simulink model "Complete_Model"  (R2023a) (continued)
== system Complete_Model/Equivalent Wind Park/300MW Eq WT3/big scope == (continued)
  To Workspace4 : ToWorkspace  VariableName=Wind_speed10  SaveFormat=Timeseries  SampleTime=-1
  To Workspace5 : ToWorkspace  VariableName=omega_m10  SaveFormat=Timeseries  SampleTime=-1
  To Workspace6 : ToWorkspace  VariableName=omega_m_opt10  SaveFormat=Timeseries  SampleTime=-1
  To Workspace7 : ToWorkspace  VariableName=omega_m_sync10  SaveFormat=Timeseries  SampleTime=-1
  To Workspace8 : ToWorkspace  VariableName=lambda10  SaveFormat=Timeseries  SampleTime=-1
  To Workspace9 : ToWorkspace  VariableName=iqr_ref10  SaveFormat=Timeseries  SampleTime=-1
  wires:
  [vqg10] -> To Workspace32/1  "vqg_mes"
  [vqg_ref10] -> To Workspace31/1  "vqg_ref"
  [iqg10] -> To Workspace30/1  "iqg_mes"
  [iqg_ref10] -> To Workspace29/1  "iqg_ref"
  [Qg_mes10] -> To Workspace28/1  "Qg_mes"
  [vdg10] -> To Workspace26/1  "vdg_mes"
  [Qg_ref10] -> To Workspace27/1  "Qg_ref"
  [vdg_ref10] -> To Workspace25/1  "vdg_ref"
  [idg10] -> To Workspace24/1  "idg_mes"
  [idg_ref10] -> To Workspace23/1  "idg_ref"
  [Vbus_mes10] -> To Workspace22/1  "Vbus_mes"
  [Vbus_ref10] -> To Workspace21/1  "Vbus_ref"
  [Ig10] -> To Workspace20/1  "Ig"
  [Ir_mes10] -> To Workspace19/1  "Ir"
  [Iout10] -> To Workspace18/1  "Iout"
  [Vout10] -> To Workspace17/1  "Vout"
  [vqr_ref10] -> To Workspace16/1  "vqr_ref"
  [idr10] -> To Workspace15/1  "idr"
  [idr_ref10] -> To Workspace14/1  "idr_ref"
  [Tr10] -> To Workspace13/1  "Tr"
  [Tr_ref10] -> To Workspace12/1  "Tr_ref_MPPT"
  [vdr_ref10] -> To Workspace11/1  "vdr_ref"
  [iqr10] -> To Workspace10/1  "iqr"
  [iqr_ref10] -> To Workspace9/1  "iqr_ref"
  [lambda10] -> To Workspace8/1  "Tip-Speed Ratio"
  [P_m10] -> To Workspace/1  "P meca"
  [omega_m10] -> To Workspace5/1  "omega_m"
  [v_wind10] -> To Workspace4/1  "Wind speed"
  [lambda10] -> External Quantities/4  "Tip-Speed Ratio"
  Gain3/1 -> Mux6/1, To Workspace6/1  "omega_m,opt"
  Divide3/1 -> Gain3/1
  Constant1/1 -> Divide3/3
  Constant2/1 -> Divide3/2
  [v_wind10] -> Divide3/1
  [idr10] -> Mux3/2  "idr"
  [Tr10] -> Mux2/2  "Tr"
  [vdr_ref10] -> Rotor Side/3  "vdr_ref"
  [iqr_ref10] -> Mux1/1  "iqr_ref"
  Mux13/1 -> Grid Side/4
  [Qg_mes10] -> Mux13/2  "Qg_mes"
  [Qg_ref10] -> Mux13/1  "Qg_ref"
  Mux12/1 -> Grid Side/6
  [vqg_ref10] -> Mux12/1  "vqg_ref"
  [vqg10] -> Mux12/2  "vqg_mes"
  Gain2/1 -> Mux9/4, To Workspace3/1  "P rotor"
  [Pout10] -> Gain6/1
  Mux9/1 -> Power/1
  [P_m10] -> Mux9/1  "P meca"
  [Pr_mes_machine10] -> Gain2/1
  Gain7/1 -> Mux9/3, To Workspace2/1  "P stator"
  [Ps_mes_machine10] -> Gain7/1
  Gain6/1 -> Mux9/2, To Workspace1/1  "P out"
  Mux11/1 -> Grid Side/3
  [vdg10] -> Mux11/2  "vdg_mes"
  [vdg_ref10] -> Mux11/1  "vdg_ref"
  [P_m10] -> Mux8/1  "P_mech"
  Constant/1 -> Mux6/3, To Workspace7/1  "synchronous rotor speed"
  [omega_m10] -> Mux6/2  "omega_m"
  [v_wind10] -> External Quantities/1  "Wind speed"
  Mux6/1 -> External Quantities/2
  Mux8/1 -> External Quantities/3
  [Pout10] -> Gain/1
  Gain/1 -> Mux8/2  "P_grid"
  [omega_m10] -> Rotor Side/1  "omega_m"
  [idg_ref10] -> Mux5/1  "idg_ref"
  Mux4/1 -> Grid Side/1
  [idg10] -> Mux5/2  "idg_mes"
  Mux5/1 -> Grid Side/2
  [Vbus_mes10] -> Mux4/2  "Vbus_mes"
  Mux7/1 -> Grid Side/5
  [Vbus_ref10] -> Mux4/1  "Vbus_ref"
  [iqg10] -> Mux7/2  "iqg_mes"
  [Ir_mes10] -> Grid Side/9  "Ir"
  [Vout10] -> Grid Side/7  "Vout"
  [iqg_ref10] -> Mux7/1  "iqg_ref"
  [Ig10] -> Grid Side/8  "Ig"
  [Ir_mes10] -> Rotor Side/9  "Ir"
  [Iout10] -> Rotor Side/8  "Iout"
  [Vout10] -> Rotor Side/7  "Vout"
  [vqr_ref10] -> Rotor Side/6  "vqr_ref"
  [idr_ref10] -> Mux3/1  "idr_ref"
  Mux3/1 -> Rotor Side/5
  Mux2/1 -> Rotor Side/4
  [Tr_ref10] -> Mux2/1  "Tr_ref_MPPT"
  Mux1/1 -> Rotor Side/2
  [iqr10] -> Mux1/2  "iqr"

== system Complete_Model/Equivalent Wind Park/300MW Eq WT4/Aerodynamic Model ==
  note: Gearbox ratio
  note: Motor convention : Generated power
      |  is negative
  note: \Omega_m blades
  note: Cp
  note: \lambda = \Omega_m R / v_{wind}
  note: C_p (\lambda)
  note: v_{wind}
  Vw : Inport
  C_p (\lambda) : Lookup_n-D  NumberOfTableDimensions=1  BreakpointsForDimension1=tab_lambda  Table=Cp  InternalRulePriority=Speed  RndMeth=Simplest
  Constant : Constant  Value=Radius
  Divide : Product  Inputs=*/
  Divide1 : Product  Inputs=**/
  Fcn2 : Fcn  Expr=0.5*rho*pi*Radius^2*u(2)^3*u(1)
  Gain : Gain  Gain=1/N
  Gain2 : Gain  Gain=n_wt_agg
  Gain4 : Gain  Gain=-1
  Mux2 : Mux  Inputs=2
  Tm : Outport
  tag scope: P_m9=global, Tm9=global, lambda9=global
  wires:
  Gain4/1 -> Divide/1
  Constant/1 -> Divide1/1
  Divide/1 -> Tm/1, [Tm9]
  [omega_m9] -> Divide/2
  Gain2/1 -> Gain4/1, [P_m9]
  Fcn2/1 -> Gain2/1
  Mux2/1 -> Fcn2/1
  C_p (\lambda)/1 -> Mux2/1
  Divide1/1 -> [lambda9], C_p (\lambda)/1  "lambda"
  Vw/1 -> Mux2/2, Divide1/3
  Gain/1 -> Divide1/2
  [omega_m9] -> Gain/1

== system Complete_Model/Equivalent Wind Park/300MW Eq WT4/Grid Side Control ==
  Vbus_ref : Inport
  Qg_ref : Inport  Port=2
  Vbus : Inport  Port=3
  Ig_mes : Inport  Port=4
  Vs_mes : Inport  Port=5
  Demux : Demux  Outputs=2
  Demux1 : Demux  Outputs=2
  Gain : Gain  Gain=Kqg
  Gain1 : Gain  Gain=Kpg
  Normalized for PWM : Gain  Gain=1/(Vbus/2)
  "PI " : Subsystem
  PI id : Subsystem
  PI iq : Subsystem
  Sum : Sum  Inputs=|++
  Vs_mes1 : InportShadow  Port=5
  abc->alpha/beta : Subsystem
  abc->alpha/beta1 : Subsystem
  alpha/beta->abc : Subsystem
  alpha/beta->d/q : Subsystem
  alpha/beta->d/q1 : Subsystem
  angle2 : Subsystem
  cancellation of coupling terms : Subsystem
  d/q->alpha/beta : Subsystem
  Vabc_ref : Outport
  tag scope: Qg_ref9=global, Vbus_ref9=global, idg9=global, idg_ref9=global, iqg9=global, iqg_ref9=global, vdg9=global, vqg9=global
  wires:
  Vs_mes1/1 -> Sum/2
  angle2/1(theta_g) -> [theta_grid9]
  [theta_grid9] -> alpha/beta->d/q1/2(theta)
  [theta_grid9] -> d/q->alpha/beta/2(theta)
  [theta_grid9] -> alpha/beta->d/q/2(theta)
  Demux/2 -> PI iq/2(meas), [iqg9]
  Demux/1 -> PI id/2(meas), [idg9]
  Vbus/1 -> "PI "/2(meas)
  Demux1/1 -> [vdg9]
  Demux1/2 -> [vqg9]
  alpha/beta->d/q1/1(d/q) -> Demux1/1
  abc->alpha/beta1/1(alpha/beta) -> alpha/beta->d/q1/1(D/Q)
  [Vg9] -> abc->alpha/beta1/1(abc)
  cancellation of coupling terms/1(out) -> d/q->alpha/beta/1(d/q)
  Sum/1 -> Normalized for PWM/1
  alpha/beta->abc/1(abc) -> Sum/1
  Qg_ref/1 -> Gain/1, [Qg_ref9]
  Gain1/1 -> PI id/1(ref), [idg_ref9]  "idg"
  "PI "/1(out) -> Gain1/1  "Pg_ref"
  Gain/1 -> [iqg_ref9], PI iq/1(ref)  "iqg"
  Vbus_ref/1 -> "PI "/1(ref), [Vbus_ref9]
  alpha/beta->d/q/1(d/q) -> Demux/1
  PI iq/1(out) -> cancellation of coupling terms/2(Vq*)
  PI id/1(out) -> cancellation of coupling terms/1(Vd*)
  Vs_mes/1 -> angle2/1(Vs_mes)
  Normalized for PWM/1 -> Vabc_ref/1
  abc->alpha/beta/1(alpha/beta) -> alpha/beta->d/q/1(D/Q)
  Ig_mes/1 -> abc->alpha/beta/1(abc)
  d/q->alpha/beta/1(D/Q) -> alpha/beta->abc/1(D/Q)

== system Complete_Model/Equivalent Wind Park/300MW Eq WT4/Power Calculations ==
  Demux{,1..3} (4 alike) : Demux  Outputs=2
  Divide : Product  Inputs=**
  Gain : Gain  Gain=3/2
  Gain1 : Gain  Gain=-1
  Gain2 : Gain  Gain=-1
  Power (3ph, Instantaneous){1..4} (4 alike) : spsPower3phInstantaneousLib/Power (3ph, Instantaneous)
  Scope{1..8,10..13} (12 alike) : Scope
  Scope9 : Scope  NumInputPorts=2
  "Second-Order " : spsSecondOrderFilterLib/Second-Order Filter  FilterType=Lowpass  Fo=17  Zeta=0.7  Ts=7.4074e-05  Initialize=off  Vac_Init="[0,  0,  60]"  Vdc_Init=0  PlotResponse=off  FreqRange=[0, 500, 1]
      settings: AttributesFormatString=f0=%<Fo>
  Second-Order 1 : spsSecondOrderFilterLib/Second-Order Filter  FilterType=Lowpass  Fo=17  Zeta=0.7  Ts=7.4074e-05  Initialize=off  Vac_Init="[0,  0,  60]"  Vdc_Init=0  PlotResponse=off  FreqRange=[0, 500, 1]
      settings: AttributesFormatString=f0=%<Fo>
  Second-Order Low-Pass Filter {1..7} (7 alike) : spsSecondOrderFilterLib/Second-Order Filter  FilterType=Lowpass  Fo=17  Zeta=0.7  Ts=7.4074e-05  Initialize=off  Vac_Init="[0,  0,  60]"  Vdc_Init=0  PlotResponse=off  FreqRange=[0, 500, 1]
      settings: AttributesFormatString=f0=%<Fo>
  Subsystem : Subsystem
  Subsystem1 : Subsystem
  Subsystem2 : Subsystem
  Subsystem3 : Subsystem
  To Workspace1 : ToWorkspace  VariableName=Iout_d9  SaveFormat=Timeseries  SampleTime=-1
  To Workspace2 : ToWorkspace  VariableName=Ig_d9  SaveFormat=Timeseries  SampleTime=-1
  To Workspace21 : ToWorkspace  VariableName=Vout_d9  SaveFormat=Timeseries  SampleTime=-1
  To Workspace3 : ToWorkspace  VariableName=Vg_d9  SaveFormat=Timeseries  SampleTime=-1
  alpha/beta->d/q : Subsystem
  alpha/beta->d/q1 : Subsystem
  alpha/beta->d/q2 : Subsystem
  alpha/beta->d/q3 : Subsystem
  angle1 : Subsystem
  angle2 : Subsystem
  tag scope: Pg_mes9=global, Pout9=global, Pr_mes_machine9=global, Ps_mes_machine9=global, Qg_mes9=global, Qout9=global, Qr_mes_machine9=global, Qs_mes_machine9=global
  wires:
  [Vg9] -> angle1/1(Vs_mes), Subsystem2/1(abc)
  Subsystem2/1(alpha/beta) -> alpha/beta->d/q2/1(D/Q)
  alpha/beta->d/q2/1(d/q) -> Demux2/1
  Demux2/1 -> To Workspace3/1, Scope10/1
  Demux2/2 -> Scope11/1
  Demux3/2 -> Scope13/1
  Demux3/1 -> Scope12/1, To Workspace2/1
  alpha/beta->d/q3/1(d/q) -> Demux3/1
  Subsystem3/1(alpha/beta) -> alpha/beta->d/q3/1(D/Q)
  angle1/1(theta_g) -> alpha/beta->d/q3/2(theta), alpha/beta->d/q2/2(theta)
  [Ig9] -> Subsystem3/1(abc)
  Gain2/1 -> Second-Order 1/1
  Gain/1 -> Gain2/1
  Gain1/1 -> Scope9/2
  Second-Order 1/1 -> Scope9/1  "Pout"
  Divide/1 -> Gain/1
  [Iout9] -> Subsystem1/1(abc)
  angle2/1(theta_g) -> alpha/beta->d/q/2(theta), alpha/beta->d/q1/2(theta)
  Subsystem1/1(alpha/beta) -> alpha/beta->d/q1/1(D/Q)
  alpha/beta->d/q1/1(d/q) -> Demux1/1
  Demux1/1 -> To Workspace1/1, Scope7/1, Divide/2
  Demux1/2 -> Scope8/1
  Demux/2 -> Scope6/1
  Demux/1 -> Scope2/1, To Workspace21/1, Divide/1
  alpha/beta->d/q/1(d/q) -> Demux/1
  Subsystem/1(alpha/beta) -> alpha/beta->d/q/1(D/Q)
  [Vout9] -> Subsystem/1(abc), angle2/1(Vs_mes)
  [Vr_mes9] -> "Power (3ph, Instantaneous)4"/1
  "Power (3ph, Instantaneous)1"/1 -> Second-Order Low-Pass Filter 7/1  "Pr"
  "Power (3ph, Instantaneous)4"/1 -> Second-Order Low-Pass Filter 5/1  "Pr"
  [Vout9] -> "Power (3ph, Instantaneous)2"/1
  "Power (3ph, Instantaneous)3"/2 -> Second-Order Low-Pass Filter 2/1
  [Vg9] -> "Power (3ph, Instantaneous)1"/1
  Second-Order Low-Pass Filter 2/1 -> [Qs_mes_machine9]  "Q_stator"
  [Vs_mes_machine9] -> "Power (3ph, Instantaneous)3"/1
  "Power (3ph, Instantaneous)2"/1 -> "Second-Order "/1
  Second-Order Low-Pass Filter 5/1 -> [Pr_mes_machine9]  "P_rotor"
  [Is_mes_machine9] -> "Power (3ph, Instantaneous)3"/2, Scope4/1
  Second-Order Low-Pass Filter 7/1 -> [Pg_mes9]  "P_rotor"
  [Ir_mes9] -> "Power (3ph, Instantaneous)4"/2, Scope5/1
  "Second-Order "/1 -> [Pout9], Gain1/1  "Pout"
  Second-Order Low-Pass Filter 6/1 -> [Qg_mes9]  "Q_rotor"
  Second-Order Low-Pass Filter 4/1 -> [Qr_mes_machine9]  "Q_rotor"
  "Power (3ph, Instantaneous)3"/1 -> Second-Order Low-Pass Filter 3/1  "Ps"
  [Ig9] -> Scope1/1, "Power (3ph, Instantaneous)1"/2
  "Power (3ph, Instantaneous)4"/2 -> Second-Order Low-Pass Filter 4/1
  [Iout9] -> "Power (3ph, Instantaneous)2"/2, Scope3/1
  "Power (3ph, Instantaneous)1"/2 -> Second-Order Low-Pass Filter 6/1
  Second-Order Low-Pass Filter 3/1 -> [Ps_mes_machine9]  "P_stator"
  Second-Order Low-Pass Filter 1/1 -> [Qout9]  "Qout"
  "Power (3ph, Instantaneous)2"/2 -> Second-Order Low-Pass Filter 1/1

== system Complete_Model/Equivalent Wind Park/300MW Eq WT4/Rotor Side Control ==
  note: Stator referred
  idr_ref : Inport
  Ir_mes : Inport  Port=2
  Vs_mes : Inport  Port=3
  theta_m_mes : Inport  Port=4
  speed_mes : Inport  Port=5
  D/Q->abc : Subsystem
  D/Q->d/q : Subsystem
  Demux : Demux  Outputs=2
  Gain : Gain  Gain=1/(-1.5*p*(Lm/Ls)*Fs)
  Gain1 : Gain  Gain=1/u
  Gain2 : Gain  Gain=1/u
  MPPT : Subsystem
  Normalized for PWM : Gain  Gain=1/(Vbus/2)
  PI id : Subsystem
  PI iq : Subsystem
  abc->alpha/beta : Subsystem
  angle : Subsystem
  cancellation of coupling terms : Subsystem
  d/q->D/Q : Subsystem
  Vabc_ref : Outport
  tag scope: Tr_ref9=global, idr9=global, idr_ref9=global, iqr9=global, iqr_ref9=global, vrabc_ref9=global
  wires:
  MPPT/1(Tm,opt) -> Gain/1, [Tr_ref9]
  d/q->D/Q/1(D/Q) -> D/Q->abc/1(D/Q)
  abc->alpha/beta/1(alpha/beta) -> D/Q->d/q/1(D/Q)
  Gain2/1 -> [vrabc_ref9], Normalized for PWM/1  "Rotor referred"
  D/Q->abc/1(abc) -> Gain2/1
  Normalized for PWM/1 -> Vabc_ref/1
  Gain1/1 -> abc->alpha/beta/1(abc)
  idr_ref/1 -> PI id/1(ref), [idr_ref9]
  Gain/1 -> PI iq/1(ref), [iqr_ref9]
  Demux/2 -> PI iq/2(meas), [iqr9]
  Demux/1 -> [idr9], PI id/2(meas)
  D/Q->d/q/1(d/q) -> Demux/1  "i_dq = rotor ref frame"
  speed_mes/1 -> MPPT/1(omega_m), cancellation of coupling terms/3(omega_m)
  cancellation of coupling terms/1(out) -> d/q->D/Q/1(d/q)  "Vdr_ref,Vqr_ref"
  PI iq/1(out) -> cancellation of coupling terms/2(Vq*)
  PI id/1(out) -> cancellation of coupling terms/1(Vd*)
  angle/1(theta_r) -> D/Q->d/q/2(theta), d/q->D/Q/2(theta)
  Vs_mes/1 -> angle/1(Vs_mes)
  theta_m_mes/1 -> angle/2(theta_m)
  Ir_mes/1 -> Gain1/1

== system Complete_Model/Equivalent Wind Park/300MW Eq WT4/Subsystem ==
  In1 : Inport
  Bus Selector : BusSelector  OutputSignals=Mechanical.Rotor angle thetam (rad),Mechanical.Rotor speed (wm),Mechanical.Electromagnetic torque Te (N*m)
  Second-Order Low-Pass Filter 3 : spsSecondOrderFilterLib/Second-Order Filter  FilterType=Lowpass  Fo=17  Zeta=0.7  Ts=7.4074e-05  Initialize=off  Vac_Init="[0,  0,  60]"  Vdc_Init=0  PlotResponse=off  FreqRange=[0, 500, 1]
      settings: AttributesFormatString=f0=%<Fo>
  tag scope: Tr9=global, omega_m9=global, theta9=global
  wires:
  Second-Order Low-Pass Filter 3/1 -> [omega_m9]
  Bus Selector/3 -> [Tr9]  "<Electromagnetic torque Te (N*m)>"
  Bus Selector/1 -> [theta9]  "<Rotor angle thetam (rad)>"
  In1/1 -> Bus Selector/1
  Bus Selector/2 -> Second-Order Low-Pass Filter 3/1  "<Rotor speed (wm)>"

== system Complete_Model/Equivalent Wind Park/300MW Eq WT4/Subsystem1 ==
  Constant4 : Constant  Value=0
  Step : Step  Time=50  After=-0.2*Ps  SampleTime=0
  Sum14 : Sum  Inputs=++|
  Out1 : Outport
  wires:
  Sum14/1 -> Out1/1
  Step/1 -> Sum14/1
  Constant4/1 -> Sum14/2

== system Complete_Model/Equivalent Wind Park/300MW Eq WT4/Subsystem2 ==
  Constant1 : Constant  Value=9
  Ramp1 : simulink/Sources/Ramp  slope=-0.4  start=13  InitialOutput=0
  Ramp2 : simulink/Sources/Ramp  slope=0.4  start=8  InitialOutput=0
  Sum1 : Sum  Inputs=++|
  Sum2 : Sum  Inputs=++|
  v_wind : Outport
  tag scope: v_wind9=global
  wires:
  Constant1/1 -> Sum2/2
  Sum2/1 -> v_wind/1, [v_wind9]
  Ramp1/1 -> Sum1/2
  Sum1/1 -> Sum2/1
  Ramp2/1 -> Sum1/1

== system Complete_Model/Equivalent Wind Park/300MW Eq WT4/Subsystem8 ==
  Constant4 : Constant  Value=0
  Step : Step  Time=55  After=Idr_rated*0.2  SampleTime=0
  Sum14 : Sum  Inputs=++|
  Out1 : Outport
  wires:
  Sum14/1 -> Out1/1
  Constant4/1 -> Sum14/2
  Step/1 -> Sum14/1

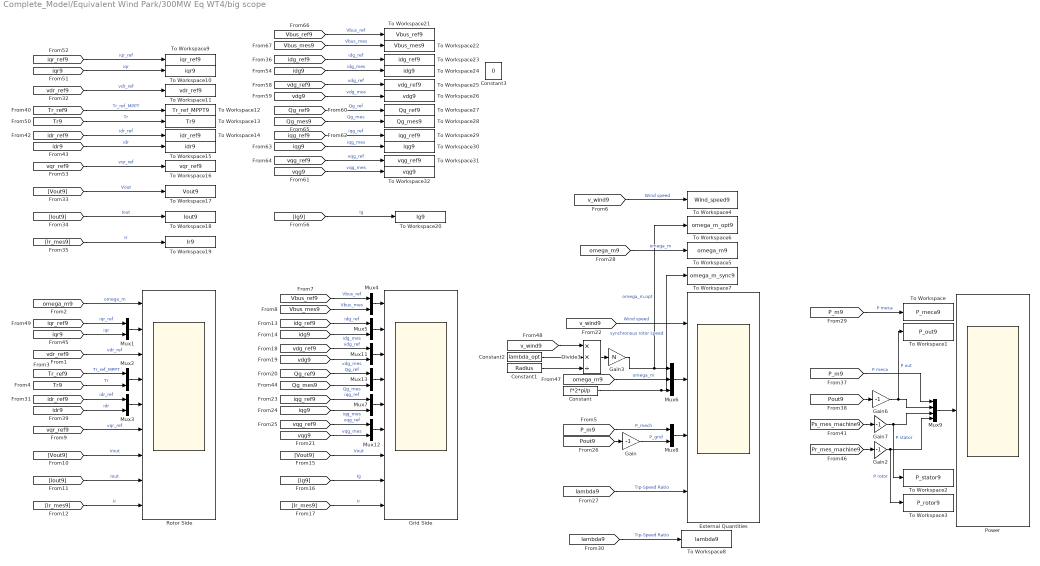
== system Complete_Model/Equivalent Wind Park/300MW Eq WT4/big scope ==
  Constant : Constant  Value=f*2*pi/p
  Constant1 : Constant  Value=Radius
  Constant2 : Constant  Value=lambda_opt
  Constant3 : Constant  Value=0
  Divide3 : Product  Inputs=**/
  External Quantities : Scope  NumInputPorts=4
  Gain{,2,6..7} (4 alike) : Gain  Gain=-1
  Gain3 : Gain  Gain=N
  Grid Side : Scope  NumInputPorts=9
  Mux{1..5,7..8,11..13} (10 alike) : Mux  Inputs=2
  Mux6 : Mux  Inputs=3
  Mux9 : Mux
  Power : Scope
  Rotor Side : Scope  NumInputPorts=9
  To Workspace : ToWorkspace  VariableName=P_meca9  SaveFormat=Timeseries  SampleTime=-1
  To Workspace1 : ToWorkspace  VariableName=P_out9  SaveFormat=Timeseries  SampleTime=-1
  To Workspace10 : ToWorkspace  VariableName=iqr9  SaveFormat=Timeseries  SampleTime=-1
  To Workspace11 : ToWorkspace  VariableName=vdr_ref9  SaveFormat=Timeseries  SampleTime=-1
  To Workspace12 : ToWorkspace  VariableName=Tr_ref_MPPT9  SaveFormat=Timeseries  SampleTime=-1
  To Workspace13 : ToWorkspace  VariableName=Tr9  SaveFormat=Timeseries  SampleTime=-1
  To Workspace14 : ToWorkspace  VariableName=idr_ref9  SaveFormat=Timeseries  SampleTime=-1
  To Workspace15 : ToWorkspace  VariableName=idr9  SaveFormat=Timeseries  SampleTime=-1
  To Workspace16 : ToWorkspace  VariableName=vqr_ref9  SaveFormat=Timeseries  SampleTime=-1
  To Workspace17 : ToWorkspace  VariableName=Vout9  SaveFormat=Timeseries  SampleTime=-1
  To Workspace18 : ToWorkspace  VariableName=Iout9  SaveFormat=Timeseries  SampleTime=-1
  To Workspace19 : ToWorkspace  VariableName=Ir9  SaveFormat=Timeseries  SampleTime=-1
  To Workspace2 : ToWorkspace  VariableName=P_stator9  SaveFormat=Timeseries  SampleTime=-1
  To Workspace20 : ToWorkspace  VariableName=Ig9  SaveFormat=Timeseries  SampleTime=-1
  To Workspace21 : ToWorkspace  VariableName=Vbus_ref9  SaveFormat=Timeseries  SampleTime=-1
  To Workspace22 : ToWorkspace  VariableName=Vbus_mes9  SaveFormat=Timeseries  SampleTime=-1
  To Workspace23 : ToWorkspace  VariableName=idg_ref9  SaveFormat=Timeseries  SampleTime=-1
  To Workspace24 : ToWorkspace  VariableName=idg9  SaveFormat=Timeseries  SampleTime=-1
  To Workspace25 : ToWorkspace  VariableName=vdg_ref9  SaveFormat=Timeseries  SampleTime=-1
  To Workspace26 : ToWorkspace  VariableName=vdg9  SaveFormat=Timeseries  SampleTime=-1
  To Workspace27 : ToWorkspace  VariableName=Qg_ref9  SaveFormat=Timeseries  SampleTime=-1
  To Workspace28 : ToWorkspace  VariableName=Qg_mes9  SaveFormat=Timeseries  SampleTime=-1
  To Workspace29 : ToWorkspace  VariableName=iqg_ref9  SaveFormat=Timeseries  SampleTime=-1
  To Workspace3 : ToWorkspace  VariableName=P_rotor9  SaveFormat=Timeseries  SampleTime=-1
  To Workspace30 : ToWorkspace  VariableName=iqg9  SaveFormat=Timeseries  SampleTime=-1
  To Workspace31 : ToWorkspace  VariableName=vqg_ref9  SaveFormat=Timeseries  SampleTime=-1
  To Workspace32 : ToWorkspace  VariableName=vqg9  SaveFormat=Timeseries  SampleTime=-1
  To Workspace4 : ToWorkspace  VariableName=Wind_speed9  SaveFormat=Timeseries  SampleTime=-1
  To Workspace5 : ToWorkspace  VariableName=omega_m9  SaveFormat=Timeseries  SampleTime=-1
  To Workspace6 : ToWorkspace  VariableName=omega_m_opt9  SaveFormat=Timeseries  SampleTime=-1
  To Workspace7 : ToWorkspace  VariableName=omega_m_sync9  SaveFormat=Timeseries  SampleTime=-1
  To Workspace8 : ToWorkspace  VariableName=lambda9  SaveFormat=Timeseries  SampleTime=-1
  To Workspace9 : ToWorkspace  VariableName=iqr_ref9  SaveFormat=Timeseries  SampleTime=-1
  wires:
  [vqg9] -> To Workspace32/1  "vqg_mes"
  [vqg_ref9] -> To Workspace31/1  "vqg_ref"
  [iqg9] -> To Workspace30/1  "iqg_mes"
  [iqg_ref9] -> To Workspace29/1  "iqg_ref"
  [Qg_mes9] -> To Workspace28/1  "Qg_mes"
  [vdg9] -> To Workspace26/1  "vdg_mes"
  [Qg_ref9] -> To Workspace27/1  "Qg_ref"
  [vdg_ref9] -> To Workspace25/1  "vdg_ref"
  [idg9] -> To Workspace24/1  "idg_mes"
  [idg_ref9] -> To Workspace23/1  "idg_ref"
  [Vbus_mes9] -> To Workspace22/1  "Vbus_mes"
  [Vbus_ref9] -> To Workspace21/1  "Vbus_ref"
  [Ig9] -> To Workspace20/1  "Ig"
  [Ir_mes9] -> To Workspace19/1  "Ir"
  [Iout9] -> To Workspace18/1  "Iout"
  [Vout9] -> To Workspace17/1  "Vout"
  [vqr_ref9] -> To Workspace16/1  "vqr_ref"
  [idr9] -> To Workspace15/1  "idr"
  [idr_ref9] -> To Workspace14/1  "idr_ref"
  [Tr9] -> To Workspace13/1  "Tr"
  [Tr_ref9] -> To Workspace12/1  "Tr_ref_MPPT"
  [vdr_ref9] -> To Workspace11/1  "vdr_ref"
  [iqr9] -> To Workspace10/1  "iqr"
  [iqr_ref9] -> To Workspace9/1  "iqr_ref"
  [lambda9] -> To Workspace8/1  "Tip-Speed Ratio"
  [P_m9] -> To Workspace/1  "P meca"
  [omega_m9] -> To Workspace5/1  "omega_m"
  [v_wind9] -> To Workspace4/1  "Wind speed"
  [lambda9] -> External Quantities/4  "Tip-Speed Ratio"
  Gain3/1 -> Mux6/1, To Workspace6/1  "omega_m,opt"
  Divide3/1 -> Gain3/1
  Constant1/1 -> Divide3/3
  Constant2/1 -> Divide3/2
  [v_wind9] -> Divide3/1
  [idr9] -> Mux3/2  "idr"
  [Tr9] -> Mux2/2  "Tr"
  [vdr_ref9] -> Rotor Side/3  "vdr_ref"
  [iqr_ref9] -> Mux1/1  "iqr_ref"
  Mux13/1 -> Grid Side/4
  [Qg_mes9] -> Mux13/2  "Qg_mes"
  [Qg_ref9] -> Mux13/1  "Qg_ref"
  Mux12/1 -> Grid Side/6
  [vqg_ref9] -> Mux12/1  "vqg_ref"
  [vqg9] -> Mux12/2  "vqg_mes"
  Gain2/1 -> Mux9/4, To Workspace3/1  "P rotor"
  [Pout9] -> Gain6/1
  Mux9/1 -> Power/1
  [P_m9] -> Mux9/1  "P meca"
  [Pr_mes_machine9] -> Gain2/1
  Gain7/1 -> Mux9/3, To Workspace2/1  "P stator"
  [Ps_mes_machine9] -> Gain7/1
  Gain6/1 -> Mux9/2, To Workspace1/1  "P out"
  Mux11/1 -> Grid Side/3
  [vdg9] -> Mux11/2  "vdg_mes"
  [vdg_ref9] -> Mux11/1  "vdg_ref"
  [P_m9] -> Mux8/1  "P_mech"
  Constant/1 -> Mux6/3, To Workspace7/1  "synchronous rotor speed"
  [omega_m9] -> Mux6/2  "omega_m"
  [v_wind9] -> External Quantities/1  "Wind speed"
  Mux6/1 -> External Quantities/2
  Mux8/1 -> External Quantities/3
  [Pout9] -> Gain/1
  Gain/1 -> Mux8/2  "P_grid"
  [omega_m9] -> Rotor Side/1  "omega_m"
  [idg_ref9] -> Mux5/1  "idg_ref"
  Mux4/1 -> Grid Side/1
  [idg9] -> Mux5/2  "idg_mes"
  Mux5/1 -> Grid Side/2
  [Vbus_mes9] -> Mux4/2  "Vbus_mes"
  Mux7/1 -> Grid Side/5
  [Vbus_ref9] -> Mux4/1  "Vbus_ref"
  [iqg9] -> Mux7/2  "iqg_mes"
  [Ir_mes9] -> Grid Side/9  "Ir"
  [Vout9] -> Grid Side/7  "Vout"
  [iqg_ref9] -> Mux7/1  "iqg_ref"
  [Ig9] -> Grid Side/8  "Ig"
  [Ir_mes9] -> Rotor Side/9  "Ir"
  [Iout9] -> Rotor Side/8  "Iout"
  [Vout9] -> Rotor Side/7  "Vout"
  [vqr_ref9] -> Rotor Side/6  "vqr_ref"
  [idr_ref9] -> Mux3/1  "idr_ref"
  Mux3/1 -> Rotor Side/5
  Mux2/1 -> Rotor Side/4
  [Tr_ref9] -> Mux2/1  "Tr_ref_MPPT"
  Mux1/1 -> Rotor Side/2
  [iqr9] -> Mux1/2  "iqr"

== system Complete_Model/Equivalent Wind Park/300MW Eq WT5/Aerodynamic Model ==
  note: Gearbox ratio
  note: Motor convention : Generated power
      |  is negative
  note: \Omega_m blades
  note: Cp
  note: \lambda = \Omega_m R / v_{wind}
  note: C_p (\lambda)
  note: v_{wind}
  Vw : Inport
  C_p (\lambda) : Lookup_n-D  NumberOfTableDimensions=1  BreakpointsForDimension1=tab_lambda  Table=Cp  InternalRulePriority=Speed  RndMeth=Simplest
  Constant : Constant  Value=Radius
  Divide : Product  Inputs=*/
  Divide1 : Product  Inputs=**/
  Fcn2 : Fcn  Expr=0.5*rho*pi*Radius^2*u(2)^3*u(1)
  Gain : Gain  Gain=1/N
  Gain2 : Gain  Gain=n_wt_agg
  Gain4 : Gain  Gain=-1
  Mux2 : Mux  Inputs=2
  Tm : Outport
  tag scope: P_m5=global, Tm5=global, lambda5=global
  wires:
  Gain4/1 -> Divide/1
  Constant/1 -> Divide1/1
  Divide/1 -> Tm/1, [Tm5]
  [omega_m5] -> Divide/2
  Gain2/1 -> Gain4/1, [P_m5]
  Fcn2/1 -> Gain2/1
  Mux2/1 -> Fcn2/1
  C_p (\lambda)/1 -> Mux2/1
  Divide1/1 -> [lambda5], C_p (\lambda)/1  "lambda"
  Vw/1 -> Mux2/2, Divide1/3
  Gain/1 -> Divide1/2
  [omega_m5] -> Gain/1

== system Complete_Model/Equivalent Wind Park/300MW Eq WT5/Grid Side Control ==
  Vbus_ref : Inport
  Qg_ref : Inport  Port=2
  Vbus : Inport  Port=3
  Ig_mes : Inport  Port=4
  Vs_mes : Inport  Port=5
  Demux : Demux  Outputs=2
  Demux1 : Demux  Outputs=2
  Gain : Gain  Gain=Kqg
  Gain1 : Gain  Gain=Kpg
  Normalized for PWM : Gain  Gain=1/(Vbus/2)
  "PI " : Subsystem
  PI id : Subsystem
  PI iq : Subsystem
  Sum : Sum  Inputs=|++
  Vs_mes1 : InportShadow  Port=5
  abc->alpha/beta : Subsystem
  abc->alpha/beta1 : Subsystem
  alpha/beta->abc : Subsystem
  alpha/beta->d/q : Subsystem
  alpha/beta->d/q1 : Subsystem
  angle2 : Subsystem
  cancellation of coupling terms : Subsystem
  d/q->alpha/beta : Subsystem
  Vabc_ref : Outport
  tag scope: Qg_ref5=global, Vbus_ref5=global, idg5=global, idg_ref5=global, iqg5=global, iqg_ref5=global, vdg5=global, vqg5=global
  wires:
  Vs_mes1/1 -> Sum/2
  angle2/1(theta_g) -> [theta_grid5]
  [theta_grid5] -> alpha/beta->d/q1/2(theta)
  [theta_grid5] -> d/q->alpha/beta/2(theta)
  [theta_grid5] -> alpha/beta->d/q/2(theta)
  Demux/2 -> PI iq/2(meas), [iqg5]
  Demux/1 -> PI id/2(meas), [idg5]
  Vbus/1 -> "PI "/2(meas)
  Demux1/1 -> [vdg5]
  Demux1/2 -> [vqg5]
  alpha/beta->d/q1/1(d/q) -> Demux1/1
  abc->alpha/beta1/1(alpha/beta) -> alpha/beta->d/q1/1(D/Q)
  [Vg5] -> abc->alpha/beta1/1(abc)
  cancellation of coupling terms/1(out) -> d/q->alpha/beta/1(d/q)
  Sum/1 -> Normalized for PWM/1
  alpha/beta->abc/1(abc) -> Sum/1
  Qg_ref/1 -> Gain/1, [Qg_ref5]
  Gain1/1 -> PI id/1(ref), [idg_ref5]  "idg"
  "PI "/1(out) -> Gain1/1  "Pg_ref"
  Gain/1 -> [iqg_ref5], PI iq/1(ref)  "iqg"
  Vbus_ref/1 -> "PI "/1(ref), [Vbus_ref5]
  alpha/beta->d/q/1(d/q) -> Demux/1
  PI iq/1(out) -> cancellation of coupling terms/2(Vq*)
  PI id/1(out) -> cancellation of coupling terms/1(Vd*)
  Vs_mes/1 -> angle2/1(Vs_mes)
  Normalized for PWM/1 -> Vabc_ref/1
  abc->alpha/beta/1(alpha/beta) -> alpha/beta->d/q/1(D/Q)
  Ig_mes/1 -> abc->alpha/beta/1(abc)
  d/q->alpha/beta/1(D/Q) -> alpha/beta->abc/1(D/Q)
